simulink model "Demo_IntelligentTrafficSigns_cs"  (Simulink 7.6)
settings: Solver=FixedStepDiscrete  SolverMode=SingleTasking  StartTime=0.0  StopTime=66  FixedStep=1/10  RelTol=1e-3
PreLoadFcn:
  | check_NUTS_defined
  | 
  | assignin('base',['model_closed_' bdroot],false);
InitFcn:
  | PreScanExperimentInitFcn(bdroot)
StopFcn:
  | PreScanExperimentStopFcn(bdroot);
  | 
undefined symbols (not defined in the file or in its folder): DiscIntNeverNeededParam, magnitude, permission, R_curve, Results, Results_20090205_104801, spare_10, spare_4, spare_5, spare_6, spare_7, spare_8, spare_9, velocity_limit, x_ini_VRML, y_ini_VRML, z_ini_VRML
libraries: simulink_extras x8, PreScanSimulinkLibrary x7, simulink x5, PreScanUsefulBlocks x3
(part 2 of 10)

== system Demo_IntelligentTrafficSigns_cs/STATE_BMW_X5_1 ==
  State : Inport  SampleTime=TimeStep
  Acceleration : Subsystem  mask(ToSensorWorld){PortName=Acceleration_BMW_X5_1, PortIndex=6, PortWidth=3, TimeStep=1/10}
      mask prompts: PortName "Port Name [-]"; PortIndex "Index [-]"; PortWidth "Port Dimensions [-]"; TimeStep "Upload Period [s]"
      mask help: Sends signal(s) from Simulink to the Sensor World
      mask Help:
      | Sends signal(s) from Simulink to the Sensor World.
      | 
      | - Port Name [-]: specifies the name of the output terminal of the Simulink block.
      | - Index [-]: specifies the start position in the output vector sent to the Sensor World
      | - Port dimensions [-]: specifies the width (number of scalars) of the output port
      | - Upload period [s]: specifies the upload period (in seconds) of the port.
      mask Display: port_label('input', 1, PortName)
  Demux : Demux  Outputs=5
  Position : Subsystem  mask(ToSensorWorld){PortName=Position_BMW_X5_1, PortIndex=0, PortWidth=3, TimeStep=1/10}
      mask prompts: PortName "Port Name [-]"; PortIndex "Index [-]"; PortWidth "Port Dimensions [-]"; TimeStep "Upload Period [s]"
      mask help: Sends signal(s) from Simulink to the Sensor World
      mask Help:
      | Sends signal(s) from Simulink to the Sensor World.
      | 
      | - Port Name [-]: specifies the name of the output terminal of the Simulink block.
      | - Index [-]: specifies the start position in the output vector sent to the Sensor World
      | - Port dimensions [-]: specifies the width (number of scalars) of the output port
      | - Upload period [s]: specifies the upload period (in seconds) of the port.
      mask Display: port_label('input', 1, PortName)
  RPY : Subsystem  mask(ToSensorWorld){PortName=RPY_BMW_X5_1, PortIndex=9, PortWidth=3, TimeStep=1/10}
      mask prompts: PortName "Port Name [-]"; PortIndex "Index [-]"; PortWidth "Port Dimensions [-]"; TimeStep "Upload Period [s]"
      mask help: Sends signal(s) from Simulink to the Sensor World
      mask Help:
      | Sends signal(s) from Simulink to the Sensor World.
      | 
      | - Port Name [-]: specifies the name of the output terminal of the Simulink block.
      | - Index [-]: specifies the start position in the output vector sent to the Sensor World
      | - Port dimensions [-]: specifies the width (number of scalars) of the output port
      | - Upload period [s]: specifies the upload period (in seconds) of the port.
      mask Display: port_label('input', 1, PortName)
  Velocity : Subsystem  mask(ToSensorWorld){PortName=Velocity_BMW_X5_1, PortIndex=3, PortWidth=3, TimeStep=1/10}
      mask prompts: PortName "Port Name [-]"; PortIndex "Index [-]"; PortWidth "Port Dimensions [-]"; TimeStep "Upload Period [s]"
      mask help: Sends signal(s) from Simulink to the Sensor World
      mask Help:
      | Sends signal(s) from Simulink to the Sensor World.
      | 
      | - Port Name [-]: specifies the name of the output terminal of the Simulink block.
      | - Index [-]: specifies the start position in the output vector sent to the Sensor World
      | - Port dimensions [-]: specifies the width (number of scalars) of the output port
      | - Upload period [s]: specifies the upload period (in seconds) of the port.
      mask Display: port_label('input', 1, PortName)
  iniBODYAngles wrtVEH [rad] : Constant  Value=[Roll0 Pitch0 0]  SampleTime=TimeStep
  iniVEHAngles wrtGLOB [rad] : Constant  Value=[0 0 Yaw0]  SampleTime=TimeStep
  iniVEHposition wrtGLOB [m] : Constant  Value=[x0 y0 z0]  SampleTime=TimeStep
  state2FullState : PreScanSimulinkLibrary/state2FullState  SystemSampleTime=-1
  velRPY : Subsystem  mask(ToSensorWorld){PortName=velRPY_BMW_X5_1, PortIndex=12, PortWidth=3, TimeStep=1/10}
      mask prompts: PortName "Port Name [-]"; PortIndex "Index [-]"; PortWidth "Port Dimensions [-]"; TimeStep "Upload Period [s]"
      mask help: Sends signal(s) from Simulink to the Sensor World
      mask Help:
      | Sends signal(s) from Simulink to the Sensor World.
      | 
      | - Port Name [-]: specifies the name of the output terminal of the Simulink block.
      | - Index [-]: specifies the start position in the output vector sent to the Sensor World
      | - Port dimensions [-]: specifies the width (number of scalars) of the output port
      | - Upload period [s]: specifies the upload period (in seconds) of the port.
      mask Display: port_label('input', 1, PortName)
  wires:
  Demux/5 -> velRPY/1(Y)
  Demux/4 -> RPY/1(Y)
  Demux/3 -> Acceleration/1(Y)
  Demux/2 -> Velocity/1(Y)
  Demux/1 -> Position/1(Y)
  State/1 -> state2FullState/1
  state2FullState/1 -> Demux/1
  iniVEHposition wrtGLOB [m]/1 -> state2FullState/2
  iniBODYAngles wrtVEH [rad]/1 -> state2FullState/3
  iniVEHAngles wrtGLOB [rad]/1 -> state2FullState/4

== system Demo_IntelligentTrafficSigns_cs/STATE_CameraTrolley ==
  State : Inport  SampleTime=TimeStep
  Acceleration : Subsystem  mask(ToSensorWorld){PortName=Acceleration_CameraTrolley, PortIndex=249, PortWidth=3, TimeStep=1/10}
      mask prompts: PortName "Port Name [-]"; PortIndex "Index [-]"; PortWidth "Port Dimensions [-]"; TimeStep "Upload Period [s]"
      mask help: Sends signal(s) from Simulink to the Sensor World
      mask Help:
      | Sends signal(s) from Simulink to the Sensor World.
      | 
      | - Port Name [-]: specifies the name of the output terminal of the Simulink block.
      | - Index [-]: specifies the start position in the output vector sent to the Sensor World
      | - Port dimensions [-]: specifies the width (number of scalars) of the output port
      | - Upload period [s]: specifies the upload period (in seconds) of the port.
      mask Display: port_label('input', 1, PortName)
  Demux : Demux  Outputs=5
  Position : Subsystem  mask(ToSensorWorld){PortName=Position_CameraTrolley, PortIndex=243, PortWidth=3, TimeStep=1/10}
      mask prompts: PortName "Port Name [-]"; PortIndex "Index [-]"; PortWidth "Port Dimensions [-]"; TimeStep "Upload Period [s]"
      mask help: Sends signal(s) from Simulink to the Sensor World
      mask Help:
      | Sends signal(s) from Simulink to the Sensor World.
      | 
      | - Port Name [-]: specifies the name of the output terminal of the Simulink block.
      | - Index [-]: specifies the start position in the output vector sent to the Sensor World
      | - Port dimensions [-]: specifies the width (number of scalars) of the output port
      | - Upload period [s]: specifies the upload period (in seconds) of the port.
      mask Display: port_label('input', 1, PortName)
  RPY : Subsystem  mask(ToSensorWorld){PortName=RPY_CameraTrolley, PortIndex=252, PortWidth=3, TimeStep=1/10}
      mask prompts: PortName "Port Name [-]"; PortIndex "Index [-]"; PortWidth "Port Dimensions [-]"; TimeStep "Upload Period [s]"
      mask help: Sends signal(s) from Simulink to the Sensor World
      mask Help:
      | Sends signal(s) from Simulink to the Sensor World.
      | 
      | - Port Name [-]: specifies the name of the output terminal of the Simulink block.
      | - Index [-]: specifies the start position in the output vector sent to the Sensor World
      | - Port dimensions [-]: specifies the width (number of scalars) of the output port
      | - Upload period [s]: specifies the upload period (in seconds) of the port.
      mask Display: port_label('input', 1, PortName)
  Velocity : Subsystem  mask(ToSensorWorld){PortName=Velocity_CameraTrolley, PortIndex=246, PortWidth=3, TimeStep=1/10}
      mask prompts: PortName "Port Name [-]"; PortIndex "Index [-]"; PortWidth "Port Dimensions [-]"; TimeStep "Upload Period [s]"
      mask help: Sends signal(s) from Simulink to the Sensor World
      mask Help:
      | Sends signal(s) from Simulink to the Sensor World.
      | 
      | - Port Name [-]: specifies the name of the output terminal of the Simulink block.
      | - Index [-]: specifies the start position in the output vector sent to the Sensor World
      | - Port dimensions [-]: specifies the width (number of scalars) of the output port
      | - Upload period [s]: specifies the upload period (in seconds) of the port.
      mask Display: port_label('input', 1, PortName)
  iniBODYAngles wrtVEH [rad] : Constant  Value=[Roll0 Pitch0 0]  SampleTime=TimeStep
  iniVEHAngles wrtGLOB [rad] : Constant  Value=[0 0 Yaw0]  SampleTime=TimeStep
  iniVEHposition wrtGLOB [m] : Constant  Value=[x0 y0 z0]  SampleTime=TimeStep
  state2FullState : PreScanSimulinkLibrary/state2FullState  SystemSampleTime=-1
  velRPY : Subsystem  mask(ToSensorWorld){PortName=velRPY_CameraTrolley, PortIndex=255, PortWidth=3, TimeStep=1/10}
      mask prompts: PortName "Port Name [-]"; PortIndex "Index [-]"; PortWidth "Port Dimensions [-]"; TimeStep "Upload Period [s]"
      mask help: Sends signal(s) from Simulink to the Sensor World
      mask Help:
      | Sends signal(s) from Simulink to the Sensor World.
      | 
      | - Port Name [-]: specifies the name of the output terminal of the Simulink block.
      | - Index [-]: specifies the start position in the output vector sent to the Sensor World
      | - Port dimensions [-]: specifies the width (number of scalars) of the output port
      | - Upload period [s]: specifies the upload period (in seconds) of the port.
      mask Display: port_label('input', 1, PortName)
  wires:
  Demux/5 -> velRPY/1(Y)
  Demux/4 -> RPY/1(Y)
  Demux/3 -> Acceleration/1(Y)
  Demux/2 -> Velocity/1(Y)
  Demux/1 -> Position/1(Y)
  State/1 -> state2FullState/1
  state2FullState/1 -> Demux/1
  iniVEHposition wrtGLOB [m]/1 -> state2FullState/2
  iniBODYAngles wrtVEH [rad]/1 -> state2FullState/3
  iniVEHAngles wrtGLOB [rad]/1 -> state2FullState/4

== system Demo_IntelligentTrafficSigns_cs/SetActuators ==
  Bus Creator : BusCreator  Inputs=1
  Data Store Read : DataStoreRead  DataStoreName=SimCoreActuators  SampleTime=TimeStep
  SendActuators : M-S-Function  FunctionName=SendActuatorsBlock  Parameters=TimeStep NrOfActuators
  dummy : Outport
  tag scope: SimCoreInfo=global
  wires:
  Bus Creator/1 -> [SimCoreInfo]  "SimCore"
  SendActuators/2 -> Bus Creator/1  "ComputationTime[s]"
  SendActuators/1 -> dummy/1
  Data Store Read/1 -> SendActuators/1

== system Demo_IntelligentTrafficSigns_cs/SimCoreInfo ==
  Bus Selector : BusSelector  OutputSignals=ComputationTime[s]
  Time : Scope
      settings: TimeRange=10  YMin=0  YMax=0.5  SaveName=ComputationTime
  wires:
  Bus Selector/1 -> Time/1  "<ComputationTime[s]>"
  [SimCoreInfo] -> Bus Selector/1

== system Demo_IntelligentTrafficSigns_cs/TS_Lcurve_R14 ==
  Ground_{1..14} (14 alike) : Ground
  Tx_Lcurve_R14_BusAssignment : BusAssignment  Tag=BusAntennaT  AssignedSignals=velocity_limit,permission,R_curve,spare_4,spare_5,spare_6,spare_7,spare_8,spare_9,spare_10
  Tx_Lcurve_R14_Mux : Subsystem  Tag=MuxAntennaT  mask(PreScan Mux Block:){Channel=1, TimeStep=1/10}
      mask prompts: TimeStep "Time Step [s]"
      settings: DeleteFcn=PreScanBlockDeleteFcn;
      mask Display:
      | fprintf('Channel: %d',Channel)
      | port_label('input', 1, 'Warning signal ID')
      | port_label('input', 2, 'Maneuvre ID')
      | port_label('input', 3, 'Vehicle Type')
      | port_label('input', 4, 'Braking Capacity')
      | port_label('input', 5, 'Vehicle Size')
      | port_label('input', 6, 'spare')
  Warning Radius curve : Constant  Value=14
  Tx_Lcurve_R14 : Outport  Tag=AntennaTOutport
  wires:
  Tx_Lcurve_R14_Mux/2(Out2) -> [Tx_Lcurve_R14]
  [Tx_Lcurve_R14] -> Tx_Lcurve_R14_BusAssignment/1
  Tx_Lcurve_R14_BusAssignment/1 -> Tx_Lcurve_R14_Mux/6(spare)
  Tx_Lcurve_R14_Mux/1(Out1) -> Tx_Lcurve_R14/1
  Warning Radius curve/1 -> Tx_Lcurve_R14_BusAssignment/4
  Ground_1/1 -> Tx_Lcurve_R14_BusAssignment/2
  Ground_2/1 -> Tx_Lcurve_R14_BusAssignment/3
  Ground_3/1 -> Tx_Lcurve_R14_BusAssignment/5
  Ground_4/1 -> Tx_Lcurve_R14_BusAssignment/6
  Ground_5/1 -> Tx_Lcurve_R14_BusAssignment/7
  Ground_6/1 -> Tx_Lcurve_R14_BusAssignment/8
  Ground_7/1 -> Tx_Lcurve_R14_BusAssignment/9
  Ground_8/1 -> Tx_Lcurve_R14_BusAssignment/10
  Ground_9/1 -> Tx_Lcurve_R14_BusAssignment/11
  Ground_10/1 -> Tx_Lcurve_R14_Mux/1(warning_signal_id)
  Ground_11/1 -> Tx_Lcurve_R14_Mux/2(maneuvre_id)
  Ground_12/1 -> Tx_Lcurve_R14_Mux/3(vehicle_type)
  Ground_13/1 -> Tx_Lcurve_R14_Mux/4(brakingcapacity)
  Ground_14/1 -> Tx_Lcurve_R14_Mux/5(vehicle_size)

== system Demo_IntelligentTrafficSigns_cs/TS_Lcurve_R25 ==
  Ground_{1..14} (14 alike) : Ground
  Tx_Lcurve_R25_BusAssignment : BusAssignment  Tag=BusAntennaT  AssignedSignals=velocity_limit,permission,R_curve,spare_4,spare_5,spare_6,spare_7,spare_8,spare_9,spare_10
  Tx_Lcurve_R25_Mux : Subsystem  Tag=MuxAntennaT  mask(PreScan Mux Block:){Channel=1, TimeStep=1/10}
      mask prompts: TimeStep "Time Step [s]"
      settings: DeleteFcn=PreScanBlockDeleteFcn;
      mask Display:
      | fprintf('Channel: %d',Channel)
      | port_label('input', 1, 'Warning signal ID')
      | port_label('input', 2, 'Maneuvre ID')
      | port_label('input', 3, 'Vehicle Type')
      | port_label('input', 4, 'Braking Capacity')
      | port_label('input', 5, 'Vehicle Size')
      | port_label('input', 6, 'spare')
  Warning Radius curve : Constant  Value=25
  Tx_Lcurve_R25 : Outport  Tag=AntennaTOutport
  wires:
  Tx_Lcurve_R25_Mux/2(Out2) -> [Tx_Lcurve_R25]
  [Tx_Lcurve_R25] -> Tx_Lcurve_R25_BusAssignment/1
  Tx_Lcurve_R25_BusAssignment/1 -> Tx_Lcurve_R25_Mux/6(spare)
  Tx_Lcurve_R25_Mux/1(Out1) -> Tx_Lcurve_R25/1
  Warning Radius curve/1 -> Tx_Lcurve_R25_BusAssignment/4
  Ground_1/1 -> Tx_Lcurve_R25_BusAssignment/2
  Ground_2/1 -> Tx_Lcurve_R25_BusAssignment/3
  Ground_3/1 -> Tx_Lcurve_R25_BusAssignment/5
  Ground_4/1 -> Tx_Lcurve_R25_BusAssignment/6
  Ground_5/1 -> Tx_Lcurve_R25_BusAssignment/7
  Ground_6/1 -> Tx_Lcurve_R25_BusAssignment/8
  Ground_7/1 -> Tx_Lcurve_R25_BusAssignment/9
  Ground_8/1 -> Tx_Lcurve_R25_BusAssignment/10
  Ground_9/1 -> Tx_Lcurve_R25_BusAssignment/11
  Ground_10/1 -> Tx_Lcurve_R25_Mux/1(warning_signal_id)
  Ground_11/1 -> Tx_Lcurve_R25_Mux/2(maneuvre_id)
  Ground_12/1 -> Tx_Lcurve_R25_Mux/3(vehicle_type)
  Ground_13/1 -> Tx_Lcurve_R25_Mux/4(brakingcapacity)
  Ground_14/1 -> Tx_Lcurve_R25_Mux/5(vehicle_size)

== system Demo_IntelligentTrafficSigns_cs/TS_Lcurve_R39 ==
  Ground_{1..14} (14 alike) : Ground
  Tx_Lcurve_R39_BusAssignment : BusAssignment  Tag=BusAntennaT  AssignedSignals=velocity_limit,permission,R_curve,spare_4,spare_5,spare_6,spare_7,spare_8,spare_9,spare_10
  Tx_Lcurve_R39_Mux : Subsystem  Tag=MuxAntennaT  mask(PreScan Mux Block:){Channel=1, TimeStep=1/10}
      mask prompts: TimeStep "Time Step [s]"
      settings: DeleteFcn=PreScanBlockDeleteFcn;
      mask Display:
      | fprintf('Channel: %d',Channel)
      | port_label('input', 1, 'Warning signal ID')
      | port_label('input', 2, 'Maneuvre ID')
      | port_label('input', 3, 'Vehicle Type')
      | port_label('input', 4, 'Braking Capacity')
      | port_label('input', 5, 'Vehicle Size')
      | port_label('input', 6, 'spare')
  Warning Radius curve : Constant  Value=39
  Tx_Lcurve_R39 : Outport  Tag=AntennaTOutport
  wires:
  Tx_Lcurve_R39_Mux/2(Out2) -> [Tx_Lcurve_R39]
  [Tx_Lcurve_R39] -> Tx_Lcurve_R39_BusAssignment/1
  Tx_Lcurve_R39_BusAssignment/1 -> Tx_Lcurve_R39_Mux/6(spare)
  Tx_Lcurve_R39_Mux/1(Out1) -> Tx_Lcurve_R39/1
  Warning Radius curve/1 -> Tx_Lcurve_R39_BusAssignment/4
  Ground_1/1 -> Tx_Lcurve_R39_BusAssignment/2
  Ground_2/1 -> Tx_Lcurve_R39_BusAssignment/3
  Ground_3/1 -> Tx_Lcurve_R39_BusAssignment/5
  Ground_4/1 -> Tx_Lcurve_R39_BusAssignment/6
  Ground_5/1 -> Tx_Lcurve_R39_BusAssignment/7
  Ground_6/1 -> Tx_Lcurve_R39_BusAssignment/8
  Ground_7/1 -> Tx_Lcurve_R39_BusAssignment/9
  Ground_8/1 -> Tx_Lcurve_R39_BusAssignment/10
  Ground_9/1 -> Tx_Lcurve_R39_BusAssignment/11
  Ground_10/1 -> Tx_Lcurve_R39_Mux/1(warning_signal_id)
  Ground_11/1 -> Tx_Lcurve_R39_Mux/2(maneuvre_id)
  Ground_12/1 -> Tx_Lcurve_R39_Mux/3(vehicle_type)
  Ground_13/1 -> Tx_Lcurve_R39_Mux/4(brakingcapacity)
  Ground_14/1 -> Tx_Lcurve_R39_Mux/5(vehicle_size)

== system Demo_IntelligentTrafficSigns_cs/TS_Lcurve_R55 ==
  Ground_{1..14} (14 alike) : Ground
  Tx_Lcurve_R55_BusAssignment : BusAssignment  Tag=BusAntennaT  AssignedSignals=velocity_limit,permission,R_curve,spare_4,spare_5,spare_6,spare_7,spare_8,spare_9,spare_10
  Tx_Lcurve_R55_Mux : Subsystem  Tag=MuxAntennaT  mask(PreScan Mux Block:){Channel=1, TimeStep=1/10}
      mask prompts: TimeStep "Time Step [s]"
      settings: DeleteFcn=PreScanBlockDeleteFcn;
      mask Display:
      | fprintf('Channel: %d',Channel)
      | port_label('input', 1, 'Warning signal ID')
      | port_label('input', 2, 'Maneuvre ID')
      | port_label('input', 3, 'Vehicle Type')
      | port_label('input', 4, 'Braking Capacity')
      | port_label('input', 5, 'Vehicle Size')
      | port_label('input', 6, 'spare')
  Warning Radius curve : Constant  Value=55
  Tx_Lcurve_R55 : Outport  Tag=AntennaTOutport
  wires:
  Tx_Lcurve_R55_Mux/2(Out2) -> [Tx_Lcurve_R55]
  [Tx_Lcurve_R55] -> Tx_Lcurve_R55_BusAssignment/1
  Tx_Lcurve_R55_BusAssignment/1 -> Tx_Lcurve_R55_Mux/6(spare)
  Tx_Lcurve_R55_Mux/1(Out1) -> Tx_Lcurve_R55/1
  Warning Radius curve/1 -> Tx_Lcurve_R55_BusAssignment/4
  Ground_1/1 -> Tx_Lcurve_R55_BusAssignment/2
  Ground_2/1 -> Tx_Lcurve_R55_BusAssignment/3
  Ground_3/1 -> Tx_Lcurve_R55_BusAssignment/5
  Ground_4/1 -> Tx_Lcurve_R55_BusAssignment/6
  Ground_5/1 -> Tx_Lcurve_R55_BusAssignment/7
  Ground_6/1 -> Tx_Lcurve_R55_BusAssignment/8
  Ground_7/1 -> Tx_Lcurve_R55_BusAssignment/9
  Ground_8/1 -> Tx_Lcurve_R55_BusAssignment/10
  Ground_9/1 -> Tx_Lcurve_R55_BusAssignment/11
  Ground_10/1 -> Tx_Lcurve_R55_Mux/1(warning_signal_id)
  Ground_11/1 -> Tx_Lcurve_R55_Mux/2(maneuvre_id)
  Ground_12/1 -> Tx_Lcurve_R55_Mux/3(vehicle_type)
  Ground_13/1 -> Tx_Lcurve_R55_Mux/4(brakingcapacity)
  Ground_14/1 -> Tx_Lcurve_R55_Mux/5(vehicle_size)

== system Demo_IntelligentTrafficSigns_cs/TS_Rcurve_R14 ==
  Ground_{1..14} (14 alike) : Ground
  Tx_Rcurve_R14_BusAssignment : BusAssignment  Tag=BusAntennaT  AssignedSignals=velocity_limit,permission,R_curve,spare_4,spare_5,spare_6,spare_7,spare_8,spare_9,spare_10
  Tx_Rcurve_R14_Mux : Subsystem  Tag=MuxAntennaT  mask(PreScan Mux Block:){Channel=1, TimeStep=1/10}
      mask prompts: TimeStep "Time Step [s]"
      settings: DeleteFcn=PreScanBlockDeleteFcn;
      mask Display:
      | fprintf('Channel: %d',Channel)
      | port_label('input', 1, 'Warning signal ID')
      | port_label('input', 2, 'Maneuvre ID')
      | port_label('input', 3, 'Vehicle Type')
      | port_label('input', 4, 'Braking Capacity')
      | port_label('input', 5, 'Vehicle Size')
      | port_label('input', 6, 'spare')
  Warning Radius curve : Constant  Value=14
  Tx_Rcurve_R14 : Outport  Tag=AntennaTOutport
  wires:
  Tx_Rcurve_R14_Mux/2(Out2) -> [Tx_Rcurve_R14]
  [Tx_Rcurve_R14] -> Tx_Rcurve_R14_BusAssignment/1
  Tx_Rcurve_R14_BusAssignment/1 -> Tx_Rcurve_R14_Mux/6(spare)
  Tx_Rcurve_R14_Mux/1(Out1) -> Tx_Rcurve_R14/1
  Warning Radius curve/1 -> Tx_Rcurve_R14_BusAssignment/4
  Ground_1/1 -> Tx_Rcurve_R14_BusAssignment/2
  Ground_2/1 -> Tx_Rcurve_R14_BusAssignment/3
  Ground_3/1 -> Tx_Rcurve_R14_BusAssignment/5
  Ground_4/1 -> Tx_Rcurve_R14_BusAssignment/6
  Ground_5/1 -> Tx_Rcurve_R14_BusAssignment/7
  Ground_6/1 -> Tx_Rcurve_R14_BusAssignment/8
  Ground_7/1 -> Tx_Rcurve_R14_BusAssignment/9
  Ground_8/1 -> Tx_Rcurve_R14_BusAssignment/10
  Ground_9/1 -> Tx_Rcurve_R14_BusAssignment/11
  Ground_10/1 -> Tx_Rcurve_R14_Mux/1(warning_signal_id)
  Ground_11/1 -> Tx_Rcurve_R14_Mux/2(maneuvre_id)
  Ground_12/1 -> Tx_Rcurve_R14_Mux/3(vehicle_type)
  Ground_13/1 -> Tx_Rcurve_R14_Mux/4(brakingcapacity)
  Ground_14/1 -> Tx_Rcurve_R14_Mux/5(vehicle_size)

== system Demo_IntelligentTrafficSigns_cs/TS_Rcurve_R25 ==
  Ground_{1..14} (14 alike) : Ground
  Tx_Rcurve_R25_BusAssignment : BusAssignment  Tag=BusAntennaT  AssignedSignals=velocity_limit,permission,R_curve,spare_4,spare_5,spare_6,spare_7,spare_8,spare_9,spare_10
  Tx_Rcurve_R25_Mux : Subsystem  Tag=MuxAntennaT  mask(PreScan Mux Block:){Channel=1, TimeStep=1/10}
      mask prompts: TimeStep "Time Step [s]"
      settings: DeleteFcn=PreScanBlockDeleteFcn;
      mask Display:
      | fprintf('Channel: %d',Channel)
      | port_label('input', 1, 'Warning signal ID')
      | port_label('input', 2, 'Maneuvre ID')
      | port_label('input', 3, 'Vehicle Type')
      | port_label('input', 4, 'Braking Capacity')
      | port_label('input', 5, 'Vehicle Size')
      | port_label('input', 6, 'spare')
  Warning Radius curve : Constant  Value=25
  Tx_Rcurve_R25 : Outport  Tag=AntennaTOutport
  wires:
  Tx_Rcurve_R25_Mux/2(Out2) -> [Tx_Rcurve_R25]
  [Tx_Rcurve_R25] -> Tx_Rcurve_R25_BusAssignment/1
  Tx_Rcurve_R25_BusAssignment/1 -> Tx_Rcurve_R25_Mux/6(spare)
  Tx_Rcurve_R25_Mux/1(Out1) -> Tx_Rcurve_R25/1
  Warning Radius curve/1 -> Tx_Rcurve_R25_BusAssignment/4
  Ground_1/1 -> Tx_Rcurve_R25_BusAssignment/2
  Ground_2/1 -> Tx_Rcurve_R25_BusAssignment/3
  Ground_3/1 -> Tx_Rcurve_R25_BusAssignment/5
  Ground_4/1 -> Tx_Rcurve_R25_BusAssignment/6
  Ground_5/1 -> Tx_Rcurve_R25_BusAssignment/7
  Ground_6/1 -> Tx_Rcurve_R25_BusAssignment/8
  Ground_7/1 -> Tx_Rcurve_R25_BusAssignment/9
  Ground_8/1 -> Tx_Rcurve_R25_BusAssignment/10
  Ground_9/1 -> Tx_Rcurve_R25_BusAssignment/11
  Ground_10/1 -> Tx_Rcurve_R25_Mux/1(warning_signal_id)
  Ground_11/1 -> Tx_Rcurve_R25_Mux/2(maneuvre_id)
  Ground_12/1 -> Tx_Rcurve_R25_Mux/3(vehicle_type)
  Ground_13/1 -> Tx_Rcurve_R25_Mux/4(brakingcapacity)
  Ground_14/1 -> Tx_Rcurve_R25_Mux/5(vehicle_size)

== system Demo_IntelligentTrafficSigns_cs/TS_Rcurve_R39 ==
  Ground_{1..14} (14 alike) : Ground
  Tx_Rcurve_R39_BusAssignment : BusAssignment  Tag=BusAntennaT  AssignedSignals=velocity_limit,permission,R_curve,spare_4,spare_5,spare_6,spare_7,spare_8,spare_9,spare_10
  Tx_Rcurve_R39_Mux : Subsystem  Tag=MuxAntennaT  mask(PreScan Mux Block:){Channel=1, TimeStep=1/10}
      mask prompts: TimeStep "Time Step [s]"
      settings: DeleteFcn=PreScanBlockDeleteFcn;
      mask Display:
      | fprintf('Channel: %d',Channel)
      | port_label('input', 1, 'Warning signal ID')
      | port_label('input', 2, 'Maneuvre ID')
      | port_label('input', 3, 'Vehicle Type')
      | port_label('input', 4, 'Braking Capacity')
      | port_label('input', 5, 'Vehicle Size')
      | port_label('input', 6, 'spare')
  Warning Radius curve : Constant  Value=39
  Tx_Rcurve_R39 : Outport  Tag=AntennaTOutport
  wires:
  Tx_Rcurve_R39_Mux/2(Out2) -> [Tx_Rcurve_R39]
  [Tx_Rcurve_R39] -> Tx_Rcurve_R39_BusAssignment/1
  Tx_Rcurve_R39_BusAssignment/1 -> Tx_Rcurve_R39_Mux/6(spare)
  Tx_Rcurve_R39_Mux/1(Out1) -> Tx_Rcurve_R39/1
  Warning Radius curve/1 -> Tx_Rcurve_R39_BusAssignment/4
  Ground_1/1 -> Tx_Rcurve_R39_BusAssignment/2
  Ground_2/1 -> Tx_Rcurve_R39_BusAssignment/3
  Ground_3/1 -> Tx_Rcurve_R39_BusAssignment/5
  Ground_4/1 -> Tx_Rcurve_R39_BusAssignment/6
  Ground_5/1 -> Tx_Rcurve_R39_BusAssignment/7
  Ground_6/1 -> Tx_Rcurve_R39_BusAssignment/8
  Ground_7/1 -> Tx_Rcurve_R39_BusAssignment/9
  Ground_8/1 -> Tx_Rcurve_R39_BusAssignment/10
  Ground_9/1 -> Tx_Rcurve_R39_BusAssignment/11
  Ground_10/1 -> Tx_Rcurve_R39_Mux/1(warning_signal_id)
  Ground_11/1 -> Tx_Rcurve_R39_Mux/2(maneuvre_id)
  Ground_12/1 -> Tx_Rcurve_R39_Mux/3(vehicle_type)
  Ground_13/1 -> Tx_Rcurve_R39_Mux/4(brakingcapacity)
  Ground_14/1 -> Tx_Rcurve_R39_Mux/5(vehicle_size)

== system Demo_IntelligentTrafficSigns_cs/TS_Rcurve_R55 ==
  Ground_{1..14} (14 alike) : Ground
  Tx_Rcurve_R55_BusAssignment : BusAssignment  Tag=BusAntennaT  AssignedSignals=velocity_limit,permission,R_curve,spare_4,spare_5,spare_6,spare_7,spare_8,spare_9,spare_10
  Tx_Rcurve_R55_Mux : Subsystem  Tag=MuxAntennaT  mask(PreScan Mux Block:){Channel=1, TimeStep=1/10}
      mask prompts: TimeStep "Time Step [s]"
      settings: DeleteFcn=PreScanBlockDeleteFcn;
      mask Display:
      | fprintf('Channel: %d',Channel)
      | port_label('input', 1, 'Warning signal ID')
      | port_label('input', 2, 'Maneuvre ID')
      | port_label('input', 3, 'Vehicle Type')
      | port_label('input', 4, 'Braking Capacity')
      | port_label('input', 5, 'Vehicle Size')
      | port_label('input', 6, 'spare')
  Warning Radius curve : Constant  Value=55
  Tx_Rcurve_R55 : Outport  Tag=AntennaTOutport
  wires:
  Tx_Rcurve_R55_Mux/2(Out2) -> [Tx_Rcurve_R55]
  [Tx_Rcurve_R55] -> Tx_Rcurve_R55_BusAssignment/1
  Tx_Rcurve_R55_BusAssignment/1 -> Tx_Rcurve_R55_Mux/6(spare)
  Tx_Rcurve_R55_Mux/1(Out1) -> Tx_Rcurve_R55/1
  Warning Radius curve/1 -> Tx_Rcurve_R55_BusAssignment/4
  Ground_1/1 -> Tx_Rcurve_R55_BusAssignment/2
  Ground_2/1 -> Tx_Rcurve_R55_BusAssignment/3
  Ground_3/1 -> Tx_Rcurve_R55_BusAssignment/5
  Ground_4/1 -> Tx_Rcurve_R55_BusAssignment/6
  Ground_5/1 -> Tx_Rcurve_R55_BusAssignment/7
  Ground_6/1 -> Tx_Rcurve_R55_BusAssignment/8
  Ground_7/1 -> Tx_Rcurve_R55_BusAssignment/9
  Ground_8/1 -> Tx_Rcurve_R55_BusAssignment/10
  Ground_9/1 -> Tx_Rcurve_R55_BusAssignment/11
  Ground_10/1 -> Tx_Rcurve_R55_Mux/1(warning_signal_id)
  Ground_11/1 -> Tx_Rcurve_R55_Mux/2(maneuvre_id)
  Ground_12/1 -> Tx_Rcurve_R55_Mux/3(vehicle_type)
  Ground_13/1 -> Tx_Rcurve_R55_Mux/4(brakingcapacity)
  Ground_14/1 -> Tx_Rcurve_R55_Mux/5(vehicle_size)

== system Demo_IntelligentTrafficSigns_cs/TS_Tcross_1 ==
  Ground_{1..14} (14 alike) : Ground
  Tx_Tcross_1_BusAssignment : BusAssignment  Tag=BusAntennaT  AssignedSignals=velocity_limit,permission,R_curve,spare_4,spare_5,spare_6,spare_7,spare_8,spare_9,spare_10
  Tx_Tcross_1_Mux : Subsystem  Tag=MuxAntennaT  mask(PreScan Mux Block:){Channel=1, TimeStep=1/10}
      mask prompts: TimeStep "Time Step [s]"
      settings: DeleteFcn=PreScanBlockDeleteFcn;
      mask Display:
      | fprintf('Channel: %d',Channel)
      | port_label('input', 1, 'Warning signal ID')
      | port_label('input', 2, 'Maneuvre ID')
      | port_label('input', 3, 'Vehicle Type')
      | port_label('input', 4, 'Braking Capacity')
      | port_label('input', 5, 'Vehicle Size')
      | port_label('input', 6, 'spare')
  Warning Radius curve : Constant  Value=8.5
  Tx_Tcross_1 : Outport  Tag=AntennaTOutport
  wires:
  Tx_Tcross_1_Mux/2(Out2) -> [Tx_Tcross_1]
  [Tx_Tcross_1] -> Tx_Tcross_1_BusAssignment/1
  Tx_Tcross_1_BusAssignment/1 -> Tx_Tcross_1_Mux/6(spare)
  Tx_Tcross_1_Mux/1(Out1) -> Tx_Tcross_1/1
  Warning Radius curve/1 -> Tx_Tcross_1_BusAssignment/4
  Ground_1/1 -> Tx_Tcross_1_BusAssignment/2
  Ground_2/1 -> Tx_Tcross_1_BusAssignment/3
  Ground_3/1 -> Tx_Tcross_1_BusAssignment/5
  Ground_4/1 -> Tx_Tcross_1_BusAssignment/6
  Ground_5/1 -> Tx_Tcross_1_BusAssignment/7
  Ground_6/1 -> Tx_Tcross_1_BusAssignment/8
  Ground_7/1 -> Tx_Tcross_1_BusAssignment/9
  Ground_8/1 -> Tx_Tcross_1_BusAssignment/10
  Ground_9/1 -> Tx_Tcross_1_BusAssignment/11
  Ground_10/1 -> Tx_Tcross_1_Mux/1(warning_signal_id)
  Ground_11/1 -> Tx_Tcross_1_Mux/2(maneuvre_id)
  Ground_12/1 -> Tx_Tcross_1_Mux/3(vehicle_type)
  Ground_13/1 -> Tx_Tcross_1_Mux/4(brakingcapacity)
  Ground_14/1 -> Tx_Tcross_1_Mux/5(vehicle_size)

== system Demo_IntelligentTrafficSigns_cs/TS_Tcross_2 ==
  Ground_{1..14} (14 alike) : Ground
  Tx_Tcross_2_BusAssignment : BusAssignment  Tag=BusAntennaT  AssignedSignals=velocity_limit,permission,R_curve,spare_4,spare_5,spare_6,spare_7,spare_8,spare_9,spare_10
  Tx_Tcross_2_Mux : Subsystem  Tag=MuxAntennaT  mask(PreScan Mux Block:){Channel=1, TimeStep=1/10}
      mask prompts: TimeStep "Time Step [s]"
      settings: DeleteFcn=PreScanBlockDeleteFcn;
      mask Display:
      | fprintf('Channel: %d',Channel)
      | port_label('input', 1, 'Warning signal ID')
      | port_label('input', 2, 'Maneuvre ID')
      | port_label('input', 3, 'Vehicle Type')
      | port_label('input', 4, 'Braking Capacity')
      | port_label('input', 5, 'Vehicle Size')
      | port_label('input', 6, 'spare')
  Warning Radius curve : Constant  Value=8.5
  Tx_Tcross_2 : Outport  Tag=AntennaTOutport
  wires:
  Tx_Tcross_2_Mux/2(Out2) -> [Tx_Tcross_2]
  [Tx_Tcross_2] -> Tx_Tcross_2_BusAssignment/1
  Tx_Tcross_2_BusAssignment/1 -> Tx_Tcross_2_Mux/6(spare)
  Tx_Tcross_2_Mux/1(Out1) -> Tx_Tcross_2/1
  Warning Radius curve/1 -> Tx_Tcross_2_BusAssignment/4
  Ground_1/1 -> Tx_Tcross_2_BusAssignment/2
  Ground_2/1 -> Tx_Tcross_2_BusAssignment/3
  Ground_3/1 -> Tx_Tcross_2_BusAssignment/5
  Ground_4/1 -> Tx_Tcross_2_BusAssignment/6
  Ground_5/1 -> Tx_Tcross_2_BusAssignment/7
  Ground_6/1 -> Tx_Tcross_2_BusAssignment/8
  Ground_7/1 -> Tx_Tcross_2_BusAssignment/9
  Ground_8/1 -> Tx_Tcross_2_BusAssignment/10
  Ground_9/1 -> Tx_Tcross_2_BusAssignment/11
  Ground_10/1 -> Tx_Tcross_2_Mux/1(warning_signal_id)
  Ground_11/1 -> Tx_Tcross_2_Mux/2(maneuvre_id)
  Ground_12/1 -> Tx_Tcross_2_Mux/3(vehicle_type)
  Ground_13/1 -> Tx_Tcross_2_Mux/4(brakingcapacity)
  Ground_14/1 -> Tx_Tcross_2_Mux/5(vehicle_size)

== system Demo_IntelligentTrafficSigns_cs/TS_Tcross_3 ==
  Ground_{1..14} (14 alike) : Ground
  Tx_Tcross_3_BusAssignment : BusAssignment  Tag=BusAntennaT  AssignedSignals=velocity_limit,permission,R_curve,spare_4,spare_5,spare_6,spare_7,spare_8,spare_9,spare_10
  Tx_Tcross_3_Mux : Subsystem  Tag=MuxAntennaT  mask(PreScan Mux Block:){Channel=1, TimeStep=1/10}
      mask prompts: TimeStep "Time Step [s]"
      settings: DeleteFcn=PreScanBlockDeleteFcn;
      mask Display:
      | fprintf('Channel: %d',Channel)
      | port_label('input', 1, 'Warning signal ID')
      | port_label('input', 2, 'Maneuvre ID')
      | port_label('input', 3, 'Vehicle Type')
      | port_label('input', 4, 'Braking Capacity')
      | port_label('input', 5, 'Vehicle Size')
      | port_label('input', 6, 'spare')
  Warning Radius curve : Constant  Value=55
  Tx_Tcross_3 : Outport  Tag=AntennaTOutport
  wires:
  Tx_Tcross_3_Mux/2(Out2) -> [Tx_Tcross_3]
  [Tx_Tcross_3] -> Tx_Tcross_3_BusAssignment/1
  Tx_Tcross_3_BusAssignment/1 -> Tx_Tcross_3_Mux/6(spare)
  Tx_Tcross_3_Mux/1(Out1) -> Tx_Tcross_3/1
  Warning Radius curve/1 -> Tx_Tcross_3_BusAssignment/4
  Ground_1/1 -> Tx_Tcross_3_BusAssignment/2
  Ground_2/1 -> Tx_Tcross_3_BusAssignment/3
  Ground_3/1 -> Tx_Tcross_3_BusAssignment/5
  Ground_4/1 -> Tx_Tcross_3_BusAssignment/6
  Ground_5/1 -> Tx_Tcross_3_BusAssignment/7
  Ground_6/1 -> Tx_Tcross_3_BusAssignment/8
  Ground_7/1 -> Tx_Tcross_3_BusAssignment/9
  Ground_8/1 -> Tx_Tcross_3_BusAssignment/10
  Ground_9/1 -> Tx_Tcross_3_BusAssignment/11
  Ground_10/1 -> Tx_Tcross_3_Mux/1(warning_signal_id)
  Ground_11/1 -> Tx_Tcross_3_Mux/2(maneuvre_id)
  Ground_12/1 -> Tx_Tcross_3_Mux/3(vehicle_type)
  Ground_13/1 -> Tx_Tcross_3_Mux/4(brakingcapacity)
  Ground_14/1 -> Tx_Tcross_3_Mux/5(vehicle_size)

== system Demo_IntelligentTrafficSigns_cs/TS_end_restrictions ==
  Ground_{1..14} (14 alike) : Ground
  Tx_end_restrictions_BusAssignment : BusAssignment  Tag=BusAntennaT  AssignedSignals=velocity_limit,permission,R_curve,spare_4,spare_5,spare_6,spare_7,spare_8,spare_9,spare_10
  Tx_end_restrictions_Mux : Subsystem  Tag=MuxAntennaT  mask(PreScan Mux Block:){Channel=1, TimeStep=1/10}
      mask prompts: TimeStep "Time Step [s]"
      settings: DeleteFcn=PreScanBlockDeleteFcn;
      mask Display:
      | fprintf('Channel: %d',Channel)
      | port_label('input', 1, 'Warning signal ID')
      | port_label('input', 2, 'Maneuvre ID')
      | port_label('input', 3, 'Vehicle Type')
      | port_label('input', 4, 'Braking Capacity')
      | port_label('input', 5, 'Vehicle Size')
      | port_label('input', 6, 'spare')
  Warning Radius curve : Constant  Value=1e6
  Tx_end_restrictions : Outport  Tag=AntennaTOutport
  wires:
  Tx_end_restrictions_Mux/2(Out2) -> [Tx_end_restrictions]
  [Tx_end_restrictions] -> Tx_end_restrictions_BusAssignment/1
  Tx_end_restrictions_BusAssignment/1 -> Tx_end_restrictions_Mux/6(spare)
  Tx_end_restrictions_Mux/1(Out1) -> Tx_end_restrictions/1
  Warning Radius curve/1 -> Tx_end_restrictions_BusAssignment/4
  Ground_1/1 -> Tx_end_restrictions_BusAssignment/2
  Ground_2/1 -> Tx_end_restrictions_BusAssignment/3
  Ground_3/1 -> Tx_end_restrictions_BusAssignment/5
  Ground_4/1 -> Tx_end_restrictions_BusAssignment/6
  Ground_5/1 -> Tx_end_restrictions_BusAssignment/7
  Ground_6/1 -> Tx_end_restrictions_BusAssignment/8
  Ground_7/1 -> Tx_end_restrictions_BusAssignment/9
  Ground_8/1 -> Tx_end_restrictions_BusAssignment/10
  Ground_9/1 -> Tx_end_restrictions_BusAssignment/11
  Ground_10/1 -> Tx_end_restrictions_Mux/1(warning_signal_id)
  Ground_11/1 -> Tx_end_restrictions_Mux/2(maneuvre_id)
  Ground_12/1 -> Tx_end_restrictions_Mux/3(vehicle_type)
  Ground_13/1 -> Tx_end_restrictions_Mux/4(brakingcapacity)
  Ground_14/1 -> Tx_end_restrictions_Mux/5(vehicle_size)

== system Demo_IntelligentTrafficSigns_cs/TS_end_restrictions_r ==
  Ground_{1..14} (14 alike) : Ground
  Tx_end_restrictions_r_BusAssignment : BusAssignment  Tag=BusAntennaT  AssignedSignals=velocity_limit,permission,R_curve,spare_4,spare_5,spare_6,spare_7,spare_8,spare_9,spare_10
  Tx_end_restrictions_r_Mux : Subsystem  Tag=MuxAntennaT  mask(PreScan Mux Block:){Channel=1, TimeStep=1/10}
      mask prompts: TimeStep "Time Step [s]"
      settings: DeleteFcn=PreScanBlockDeleteFcn;
      mask Display:
      | fprintf('Channel: %d',Channel)
      | port_label('input', 1, 'Warning signal ID')
      | port_label('input', 2, 'Maneuvre ID')
      | port_label('input', 3, 'Vehicle Type')
      | port_label('input', 4, 'Braking Capacity')
      | port_label('input', 5, 'Vehicle Size')
      | port_label('input', 6, 'spare')
  Warning Radius curve : Constant  Value=1e6
  Tx_end_restrictions_r : Outport  Tag=AntennaTOutport
  wires:
  Tx_end_restrictions_r_Mux/2(Out2) -> [Tx_end_restrictions_r]
  [Tx_end_restrictions_r] -> Tx_end_restrictions_r_BusAssignment/1
  Tx_end_restrictions_r_BusAssignment/1 -> Tx_end_restrictions_r_Mux/6(spare)
  Tx_end_restrictions_r_Mux/1(Out1) -> Tx_end_restrictions_r/1
  Warning Radius curve/1 -> Tx_end_restrictions_r_BusAssignment/4
  Ground_1/1 -> Tx_end_restrictions_r_BusAssignment/2
  Ground_2/1 -> Tx_end_restrictions_r_BusAssignment/3
  Ground_3/1 -> Tx_end_restrictions_r_BusAssignment/5
  Ground_4/1 -> Tx_end_restrictions_r_BusAssignment/6
  Ground_5/1 -> Tx_end_restrictions_r_BusAssignment/7
  Ground_6/1 -> Tx_end_restrictions_r_BusAssignment/8
  Ground_7/1 -> Tx_end_restrictions_r_BusAssignment/9
  Ground_8/1 -> Tx_end_restrictions_r_BusAssignment/10
  Ground_9/1 -> Tx_end_restrictions_r_BusAssignment/11
  Ground_10/1 -> Tx_end_restrictions_r_Mux/1(warning_signal_id)
  Ground_11/1 -> Tx_end_restrictions_r_Mux/2(maneuvre_id)
  Ground_12/1 -> Tx_end_restrictions_r_Mux/3(vehicle_type)
  Ground_13/1 -> Tx_end_restrictions_r_Mux/4(brakingcapacity)
  Ground_14/1 -> Tx_end_restrictions_r_Mux/5(vehicle_size)

== system Demo_IntelligentTrafficSigns_cs/TS_no_entry ==
  Ground_{1..14} (14 alike) : Ground
  Limited Acces : Constant
  Tx_no_entry_BusAssignment : BusAssignment  Tag=BusAntennaT  AssignedSignals=velocity_limit,permission,R_curve,spare_4,spare_5,spare_6,spare_7,spare_8,spare_9,spare_10
  Tx_no_entry_Mux : Subsystem  Tag=MuxAntennaT  mask(PreScan Mux Block:){Channel=1, TimeStep=1/10}
      mask prompts: TimeStep "Time Step [s]"
      settings: DeleteFcn=PreScanBlockDeleteFcn;
      mask Display:
      | fprintf('Channel: %d',Channel)
      | port_label('input', 1, 'Warning signal ID')
      | port_label('input', 2, 'Maneuvre ID')
      | port_label('input', 3, 'Vehicle Type')
      | port_label('input', 4, 'Braking Capacity')
      | port_label('input', 5, 'Vehicle Size')
      | port_label('input', 6, 'spare')
  Tx_no_entry : Outport  Tag=AntennaTOutport
  wires:
  Tx_no_entry_Mux/2(Out2) -> [Tx_no_entry]
  [Tx_no_entry] -> Tx_no_entry_BusAssignment/1
  Tx_no_entry_BusAssignment/1 -> Tx_no_entry_Mux/6(spare)
  Tx_no_entry_Mux/1(Out1) -> Tx_no_entry/1
  Limited Acces/1 -> Tx_no_entry_BusAssignment/3
  Ground_1/1 -> Tx_no_entry_BusAssignment/2
  Ground_2/1 -> Tx_no_entry_BusAssignment/4
  Ground_3/1 -> Tx_no_entry_BusAssignment/5
  Ground_4/1 -> Tx_no_entry_BusAssignment/6
  Ground_5/1 -> Tx_no_entry_BusAssignment/7
  Ground_6/1 -> Tx_no_entry_BusAssignment/8
  Ground_7/1 -> Tx_no_entry_BusAssignment/9
  Ground_8/1 -> Tx_no_entry_BusAssignment/10
  Ground_9/1 -> Tx_no_entry_BusAssignment/11
  Ground_10/1 -> Tx_no_entry_Mux/1(warning_signal_id)
  Ground_11/1 -> Tx_no_entry_Mux/2(maneuvre_id)
  Ground_12/1 -> Tx_no_entry_Mux/3(vehicle_type)
  Ground_13/1 -> Tx_no_entry_Mux/4(brakingcapacity)
  Ground_14/1 -> Tx_no_entry_Mux/5(vehicle_size)

== system Demo_IntelligentTrafficSigns_cs/TS_v50_1 ==
  Ground_{1..14} (14 alike) : Ground
  Regulation Velocity [kmh-1] : Constant  Value=50
  Tx_v50_1_BusAssignment : BusAssignment  Tag=BusAntennaT  AssignedSignals=velocity_limit,permission,R_curve,spare_4,spare_5,spare_6,spare_7,spare_8,spare_9,spare_10
  Tx_v50_1_Mux : Subsystem  Tag=MuxAntennaT  mask(PreScan Mux Block:){Channel=1, TimeStep=1/10}
      mask prompts: TimeStep "Time Step [s]"
      settings: DeleteFcn=PreScanBlockDeleteFcn;
      mask Display:
      | fprintf('Channel: %d',Channel)
      | port_label('input', 1, 'Warning signal ID')
      | port_label('input', 2, 'Maneuvre ID')
      | port_label('input', 3, 'Vehicle Type')
      | port_label('input', 4, 'Braking Capacity')
      | port_label('input', 5, 'Vehicle Size')
      | port_label('input', 6, 'spare')
  Tx_v50_1 : Outport  Tag=AntennaTOutport
  wires:
  Tx_v50_1_Mux/2(Out2) -> [Tx_v50_1]
  [Tx_v50_1] -> Tx_v50_1_BusAssignment/1
  Tx_v50_1_BusAssignment/1 -> Tx_v50_1_Mux/6(spare)
  Tx_v50_1_Mux/1(Out1) -> Tx_v50_1/1
  Regulation Velocity [kmh-1]/1 -> Tx_v50_1_BusAssignment/2
  Ground_1/1 -> Tx_v50_1_BusAssignment/3
  Ground_2/1 -> Tx_v50_1_BusAssignment/4
  Ground_3/1 -> Tx_v50_1_BusAssignment/5
  Ground_4/1 -> Tx_v50_1_BusAssignment/6
  Ground_5/1 -> Tx_v50_1_BusAssignment/7
  Ground_6/1 -> Tx_v50_1_BusAssignment/8
  Ground_7/1 -> Tx_v50_1_BusAssignment/9
  Ground_8/1 -> Tx_v50_1_BusAssignment/10
  Ground_9/1 -> Tx_v50_1_BusAssignment/11
  Ground_10/1 -> Tx_v50_1_Mux/1(warning_signal_id)
  Ground_11/1 -> Tx_v50_1_Mux/2(maneuvre_id)
  Ground_12/1 -> Tx_v50_1_Mux/3(vehicle_type)
  Ground_13/1 -> Tx_v50_1_Mux/4(brakingcapacity)
  Ground_14/1 -> Tx_v50_1_Mux/5(vehicle_size)

== system Demo_IntelligentTrafficSigns_cs/TS_v50_2 ==
  Ground_{1..14} (14 alike) : Ground
  Regulation Velocity [kmh-1] : Constant  Value=50
  Tx_v50_2_BusAssignment : BusAssignment  Tag=BusAntennaT  AssignedSignals=velocity_limit,permission,R_curve,spare_4,spare_5,spare_6,spare_7,spare_8,spare_9,spare_10
  Tx_v50_2_Mux : Subsystem  Tag=MuxAntennaT  mask(PreScan Mux Block:){Channel=1, TimeStep=1/10}
      mask prompts: TimeStep "Time Step [s]"
      settings: DeleteFcn=PreScanBlockDeleteFcn;
      mask Display:
      | fprintf('Channel: %d',Channel)
      | port_label('input', 1, 'Warning signal ID')
      | port_label('input', 2, 'Maneuvre ID')
      | port_label('input', 3, 'Vehicle Type')
      | port_label('input', 4, 'Braking Capacity')
      | port_label('input', 5, 'Vehicle Size')
      | port_label('input', 6, 'spare')
  Tx_v50_2 : Outport  Tag=AntennaTOutport
  wires:
  Tx_v50_2_Mux/2(Out2) -> [Tx_v50_2]
  [Tx_v50_2] -> Tx_v50_2_BusAssignment/1
  Tx_v50_2_BusAssignment/1 -> Tx_v50_2_Mux/6(spare)
  Tx_v50_2_Mux/1(Out1) -> Tx_v50_2/1
  Regulation Velocity [kmh-1]/1 -> Tx_v50_2_BusAssignment/2
  Ground_1/1 -> Tx_v50_2_BusAssignment/3
  Ground_2/1 -> Tx_v50_2_BusAssignment/4
  Ground_3/1 -> Tx_v50_2_BusAssignment/5
  Ground_4/1 -> Tx_v50_2_BusAssignment/6
  Ground_5/1 -> Tx_v50_2_BusAssignment/7
  Ground_6/1 -> Tx_v50_2_BusAssignment/8
  Ground_7/1 -> Tx_v50_2_BusAssignment/9
  Ground_8/1 -> Tx_v50_2_BusAssignment/10
  Ground_9/1 -> Tx_v50_2_BusAssignment/11
  Ground_10/1 -> Tx_v50_2_Mux/1(warning_signal_id)
  Ground_11/1 -> Tx_v50_2_Mux/2(maneuvre_id)
  Ground_12/1 -> Tx_v50_2_Mux/3(vehicle_type)
  Ground_13/1 -> Tx_v50_2_Mux/4(brakingcapacity)
  Ground_14/1 -> Tx_v50_2_Mux/5(vehicle_size)

== system Demo_IntelligentTrafficSigns_cs/TS_v50_3 ==
  Ground_{1..14} (14 alike) : Ground
  Regulation Velocity [kmh-1] : Constant  Value=50
  Tx_v50_3_BusAssignment : BusAssignment  Tag=BusAntennaT  AssignedSignals=velocity_limit,permission,R_curve,spare_4,spare_5,spare_6,spare_7,spare_8,spare_9,spare_10
  Tx_v50_3_Mux : Subsystem  Tag=MuxAntennaT  mask(PreScan Mux Block:){Channel=1, TimeStep=1/10}
      mask prompts: TimeStep "Time Step [s]"
      settings: DeleteFcn=PreScanBlockDeleteFcn;
      mask Display:
      | fprintf('Channel: %d',Channel)
      | port_label('input', 1, 'Warning signal ID')
      | port_label('input', 2, 'Maneuvre ID')
      | port_label('input', 3, 'Vehicle Type')
      | port_label('input', 4, 'Braking Capacity')
      | port_label('input', 5, 'Vehicle Size')
      | port_label('input', 6, 'spare')
  Tx_v50_3 : Outport  Tag=AntennaTOutport
  wires:
  Tx_v50_3_Mux/2(Out2) -> [Tx_v50_3]
  [Tx_v50_3] -> Tx_v50_3_BusAssignment/1
  Tx_v50_3_BusAssignment/1 -> Tx_v50_3_Mux/6(spare)
  Tx_v50_3_Mux/1(Out1) -> Tx_v50_3/1
  Regulation Velocity [kmh-1]/1 -> Tx_v50_3_BusAssignment/2
  Ground_1/1 -> Tx_v50_3_BusAssignment/3
  Ground_2/1 -> Tx_v50_3_BusAssignment/4
  Ground_3/1 -> Tx_v50_3_BusAssignment/5
  Ground_4/1 -> Tx_v50_3_BusAssignment/6
  Ground_5/1 -> Tx_v50_3_BusAssignment/7
  Ground_6/1 -> Tx_v50_3_BusAssignment/8
  Ground_7/1 -> Tx_v50_3_BusAssignment/9
  Ground_8/1 -> Tx_v50_3_BusAssignment/10
  Ground_9/1 -> Tx_v50_3_BusAssignment/11
  Ground_10/1 -> Tx_v50_3_Mux/1(warning_signal_id)
  Ground_11/1 -> Tx_v50_3_Mux/2(maneuvre_id)
  Ground_12/1 -> Tx_v50_3_Mux/3(vehicle_type)
  Ground_13/1 -> Tx_v50_3_Mux/4(brakingcapacity)
  Ground_14/1 -> Tx_v50_3_Mux/5(vehicle_size)

== system Demo_IntelligentTrafficSigns_cs/TS_v80_1 ==
  Ground_{1..14} (14 alike) : Ground
  Regulation Velocity [kmh-1] : Constant  Value=80
  Tx_v80_1_BusAssignment : BusAssignment  Tag=BusAntennaT  AssignedSignals=velocity_limit,permission,R_curve,spare_4,spare_5,spare_6,spare_7,spare_8,spare_9,spare_10
  Tx_v80_1_Mux : Subsystem  Tag=MuxAntennaT  mask(PreScan Mux Block:){Channel=1, TimeStep=1/10}
      mask prompts: TimeStep "Time Step [s]"
      settings: DeleteFcn=PreScanBlockDeleteFcn;
      mask Display:
      | fprintf('Channel: %d',Channel)
      | port_label('input', 1, 'Warning signal ID')
      | port_label('input', 2, 'Maneuvre ID')
      | port_label('input', 3, 'Vehicle Type')
      | port_label('input', 4, 'Braking Capacity')
      | port_label('input', 5, 'Vehicle Size')
      | port_label('input', 6, 'spare')
  Tx_v80_1 : Outport  Tag=AntennaTOutport
  wires:
  Tx_v80_1_Mux/2(Out2) -> [Tx_v80_1]
  [Tx_v80_1] -> Tx_v80_1_BusAssignment/1
  Tx_v80_1_BusAssignment/1 -> Tx_v80_1_Mux/6(spare)
  Tx_v80_1_Mux/1(Out1) -> Tx_v80_1/1
  Regulation Velocity [kmh-1]/1 -> Tx_v80_1_BusAssignment/2
  Ground_1/1 -> Tx_v80_1_BusAssignment/3
  Ground_2/1 -> Tx_v80_1_BusAssignment/4
  Ground_3/1 -> Tx_v80_1_BusAssignment/5
  Ground_4/1 -> Tx_v80_1_BusAssignment/6
  Ground_5/1 -> Tx_v80_1_BusAssignment/7
  Ground_6/1 -> Tx_v80_1_BusAssignment/8
  Ground_7/1 -> Tx_v80_1_BusAssignment/9
  Ground_8/1 -> Tx_v80_1_BusAssignment/10
  Ground_9/1 -> Tx_v80_1_BusAssignment/11
  Ground_10/1 -> Tx_v80_1_Mux/1(warning_signal_id)
  Ground_11/1 -> Tx_v80_1_Mux/2(maneuvre_id)
  Ground_12/1 -> Tx_v80_1_Mux/3(vehicle_type)
  Ground_13/1 -> Tx_v80_1_Mux/4(brakingcapacity)
  Ground_14/1 -> Tx_v80_1_Mux/5(vehicle_size)

== system Demo_IntelligentTrafficSigns_cs/TS_v80_3 ==
  Ground_{1..14} (14 alike) : Ground
  Regulation Velocity [kmh-1] : Constant  Value=80
  Tx_v80_3_BusAssignment : BusAssignment  Tag=BusAntennaT  AssignedSignals=velocity_limit,permission,R_curve,spare_4,spare_5,spare_6,spare_7,spare_8,spare_9,spare_10
  Tx_v80_3_Mux : Subsystem  Tag=MuxAntennaT  mask(PreScan Mux Block:){Channel=1, TimeStep=1/10}
      mask prompts: TimeStep "Time Step [s]"
      settings: DeleteFcn=PreScanBlockDeleteFcn;
      mask Display:
      | fprintf('Channel: %d',Channel)
      | port_label('input', 1, 'Warning signal ID')
      | port_label('input', 2, 'Maneuvre ID')
      | port_label('input', 3, 'Vehicle Type')
      | port_label('input', 4, 'Braking Capacity')
      | port_label('input', 5, 'Vehicle Size')
      | port_label('input', 6, 'spare')
  Tx_v80_3 : Outport  Tag=AntennaTOutport
  wires:
  Tx_v80_3_Mux/2(Out2) -> [Tx_v80_3]
  [Tx_v80_3] -> Tx_v80_3_BusAssignment/1
  Tx_v80_3_BusAssignment/1 -> Tx_v80_3_Mux/6(spare)
  Tx_v80_3_Mux/1(Out1) -> Tx_v80_3/1
  Regulation Velocity [kmh-1]/1 -> Tx_v80_3_BusAssignment/2
  Ground_1/1 -> Tx_v80_3_BusAssignment/3
  Ground_2/1 -> Tx_v80_3_BusAssignment/4
  Ground_3/1 -> Tx_v80_3_BusAssignment/5
  Ground_4/1 -> Tx_v80_3_BusAssignment/6
  Ground_5/1 -> Tx_v80_3_BusAssignment/7
  Ground_6/1 -> Tx_v80_3_BusAssignment/8
  Ground_7/1 -> Tx_v80_3_BusAssignment/9
  Ground_8/1 -> Tx_v80_3_BusAssignment/10
  Ground_9/1 -> Tx_v80_3_BusAssignment/11
  Ground_10/1 -> Tx_v80_3_Mux/1(warning_signal_id)
  Ground_11/1 -> Tx_v80_3_Mux/2(maneuvre_id)
  Ground_12/1 -> Tx_v80_3_Mux/3(vehicle_type)
  Ground_13/1 -> Tx_v80_3_Mux/4(brakingcapacity)
  Ground_14/1 -> Tx_v80_3_Mux/5(vehicle_size)

== system Demo_IntelligentTrafficSigns_cs/TS_v80_4 ==
  Ground_{1..13} (13 alike) : Ground
  Regulation Velocity [kmh-1] : Constant  Value=80
  Tx_v80_4_BusAssignment : BusAssignment  Tag=BusAntennaT  AssignedSignals=velocity_limit,permission,R_curve,spare_4,spare_5,spare_6,spare_7,spare_8,spare_9,spare_10
  Tx_v80_4_Mux : Subsystem  Tag=MuxAntennaT  mask(PreScan Mux Block:){Channel=1, TimeStep=1/10}
      mask prompts: TimeStep "Time Step [s]"
      settings: DeleteFcn=PreScanBlockDeleteFcn;
      mask Display:
      | fprintf('Channel: %d',Channel)
      | port_label('input', 1, 'Warning signal ID')
      | port_label('input', 2, 'Maneuvre ID')
      | port_label('input', 3, 'Vehicle Type')
      | port_label('input', 4, 'Braking Capacity')
      | port_label('input', 5, 'Vehicle Size')
      | port_label('input', 6, 'spare')
  Warning Radius curve : Constant  Value=1e6
  Tx_v80_4 : Outport  Tag=AntennaTOutport
  wires:
  Tx_v80_4_Mux/2(Out2) -> [Tx_v80_4]
  [Tx_v80_4] -> Tx_v80_4_BusAssignment/1
  Tx_v80_4_BusAssignment/1 -> Tx_v80_4_Mux/6(spare)
  Tx_v80_4_Mux/1(Out1) -> Tx_v80_4/1
  Regulation Velocity [kmh-1]/1 -> Tx_v80_4_Mux/5(vehicle_size)
  Warning Radius curve/1 -> Tx_v80_4_BusAssignment/4
  Ground_1/1 -> Tx_v80_4_BusAssignment/2
  Ground_2/1 -> Tx_v80_4_BusAssignment/3
  Ground_3/1 -> Tx_v80_4_BusAssignment/5
  Ground_4/1 -> Tx_v80_4_BusAssignment/6
  Ground_5/1 -> Tx_v80_4_BusAssignment/7
  Ground_6/1 -> Tx_v80_4_BusAssignment/8
  Ground_7/1 -> Tx_v80_4_BusAssignment/9
  Ground_8/1 -> Tx_v80_4_BusAssignment/10
  Ground_9/1 -> Tx_v80_4_BusAssignment/11
  Ground_10/1 -> Tx_v80_4_Mux/1(warning_signal_id)
  Ground_11/1 -> Tx_v80_4_Mux/2(maneuvre_id)
  Ground_12/1 -> Tx_v80_4_Mux/3(vehicle_type)
  Ground_13/1 -> Tx_v80_4_Mux/4(brakingcapacity)

== system Demo_IntelligentTrafficSigns_cs/Tx_Lcurve_R14 ==
  Y : Inport
  Assignment : Assignment  IndexMode=Zero-based  SampleTime=TimeStep  IndexOptions=Starting index (dialog)  Indices=PortIndex  OutputSizes=[1 1]
  Data Store Read : DataStoreRead  DataStoreName=SimCoreActuators  SampleTime=TimeStep
  Data Store Write : DataStoreWrite  DataStoreName=SimCoreActuators
  wires:
  Assignment/1 -> Data Store Write/1
  Data Store Read/1 -> Assignment/1
  Y/1 -> Assignment/2

== system Demo_IntelligentTrafficSigns_cs/Tx_Lcurve_R25 ==
  Y : Inport
  Assignment : Assignment  IndexMode=Zero-based  SampleTime=TimeStep  IndexOptions=Starting index (dialog)  Indices=PortIndex  OutputSizes=[1 1]
  Data Store Read : DataStoreRead  DataStoreName=SimCoreActuators  SampleTime=TimeStep
  Data Store Write : DataStoreWrite  DataStoreName=SimCoreActuators
  wires:
  Assignment/1 -> Data Store Write/1
  Data Store Read/1 -> Assignment/1
  Y/1 -> Assignment/2

== system Demo_IntelligentTrafficSigns_cs/Tx_Lcurve_R39 ==
  Y : Inport
  Assignment : Assignment  IndexMode=Zero-based  SampleTime=TimeStep  IndexOptions=Starting index (dialog)  Indices=PortIndex  OutputSizes=[1 1]
  Data Store Read : DataStoreRead  DataStoreName=SimCoreActuators  SampleTime=TimeStep
  Data Store Write : DataStoreWrite  DataStoreName=SimCoreActuators
  wires:
  Assignment/1 -> Data Store Write/1
  Data Store Read/1 -> Assignment/1
  Y/1 -> Assignment/2
